simulink library "Renault_Library"  (Simulink 8.2)
settings: Solver=ode3  StartTime=0.0  StopTime=10.0  FixedStep=0.020  RelTol=1e-3
undefined symbols (not defined in the file or in its folder): ABC, AssystemProposal, b, chkval, CoeffA_C, CoeffB_C, CoeffMean_C, ConfTime_C, DiagDelay_C, DividePercent, FailureConfActive_C, FallingEdgeDuration_C, HighLevelThresholdVoltage_C, HighLevelVoltage_C, HighLevelVoltageTunable_C, InitValue_C, InputVoltRangeMax_C, InputVoltRangeMin_C, LowActiveContact_C, LowLevelThresholdVoltage_C, LowLevelVoltage_C, MaxOperatingVoltage_C, MinOperatingVoltage_C, mV2V_conversion, OUT1, OutOfRangeHigh_C, OutOfRangeLow_C, PinSupplySaturationMax, PinSupplySaturationMin, Res1Value_C, Res2Value_C, Res3Value_C, RisingEdgeDuration_C, RSA_CANSignalFailMonitoring, SampleTime_C, service14Table, service19Table, service22Table, service24Table, service28Table, service2ETable, service2FTable, service31Table, service87Table, SizeXStateDID2FTable, SizeXTable14, SizeXTable19, SizeXTable22, SizeXTable24, SizeXTable28, SizeXTable2E, SizeXTable2F, SizeXTable31, SizeXTable87, SizeYStateDID2FTable, SizeYTable14, SizeYTable19, SizeYTable22, SizeYTable24, SizeYTable28 ... (+22)
undefined symbols (not defined in the file): BatteryVoltage_ActivationThreshold_C, FrameStatus_AbsentValue_C, FrameStatus_ConfAbsentValue_C, MemThreshold_C, NAMEOFTHEMODEL_SAMPLE_TIME_CST, NbAbs1_C, NbConfAbs1_C, NbConfPres1_C, NbPres1_C, NOMDUSIGNAL_FaultConfTime_C, NOMDUSIGNAL_FaultRehabTime_C, NOMDUSIGNAL_switchvalue_C, NOMDUSIGNAL_unavailablevalue_C, PeriodFrame1_C, ResetTime_C, WakeUpTime_C
libraries: Renault_Library x112, simulink x75
(part 17 of 32)

== system Renault_Library/Services ECU/Diag/RSA_DiagnosticManagement_v02.00/DIAGNOSTIC_SANCTION_TOP/Service19 ==
  REQ_DATA_NUMBER : Inport
  DIAG_BUFFER_REQUEST : Inport  Port=2
  SESSION_ACTIVE : Inport  Port=3
  Trigger : TriggerPort
  Check_Lenght_Message_Service19 : Subsystem
  Check_Message_Service19 : Stateflow chart  TreatAsAtomicUnit=on
  Parameter_Valid_Service19 : Subsystem
  SubFunction_In_Active_Session_Service19 : Subsystem
  SubFunction_Supported_Service19 : Subsystem
  NEGATIVE_RES_CODE : Outport
  SUPPRESS_POS_RES : Outport  Port=2
  SEND_RES_MESSAGE : Outport  Port=3
  wires:
  REQ_DATA_NUMBER/1 -> Check_Lenght_Message_Service19/1(REQ_DATA_NUMBER)
  Check_Lenght_Message_Service19/1(LENGTH_MESSAGE_CORRECT) -> Check_Message_Service19/1(LENGTH_MESSAGE_CORRECT)
  DIAG_BUFFER_REQUEST/1 -> SubFunction_In_Active_Session_Service19/2(DIAG_BUFFER_REQUEST), Parameter_Valid_Service19/1(DIAG_BUFFER_REQUEST), SubFunction_Supported_Service19/1(DIAG_BUFFER_REQUEST)
  SubFunction_Supported_Service19/1(SUBFUNCTION_SUPPORTED) -> Check_Message_Service19/4(SUBFUNCTION_SUPPORTED)
  SubFunction_Supported_Service19/2(SUPPRESS_POS_RES) -> SUPPRESS_POS_RES/1
  SESSION_ACTIVE/1 -> SubFunction_In_Active_Session_Service19/1(SESSION)
  SubFunction_In_Active_Session_Service19/1(SUBFUNCTION_SUPPORTED_IN_ACTIVE_SESSION) -> Check_Message_Service19/2(SUBFUNCTION_SUPPORTED_IN_ACTIVE_SESSION)
  Parameter_Valid_Service19/1(PARAMETER_VALID) -> Check_Message_Service19/3(PARAMETER_VALID)
  Check_Message_Service19/1(NEGATIVE_RES_CODE) -> NEGATIVE_RES_CODE/1
  Check_Message_Service19/2(SEND_RES_MESSAGE) -> SEND_RES_MESSAGE/1

== system Renault_Library/Services ECU/Diag/RSA_DiagnosticManagement_v02.00/DIAGNOSTIC_SANCTION_TOP/Service22 ==
  REQ_DATA_NUMBER : Inport
  DIAG_BUFFER_REQUEST : Inport  Port=2
  SESSION_ACTIVE : Inport  Port=3
  Trigger : TriggerPort
  Check_Lenght_Message_Response_Service22 : Subsystem
  Check_Lenght_Message_Service22 : Subsystem
  Check_Message_Service22 : Stateflow chart  TreatAsAtomicUnit=on
  Constant : Constant  Value=true  OutDataTypeStr=boolean  SampleTime=-1
  Parameter_Valid_Service22 : Subsystem
  NEGATIVE_RES_CODE : Outport
  LIST_DID_SERVICE_22 : Outport  Port=2  PortDimensions=[2046 3]
  SEND_RES_MESSAGE : Outport  Port=3
  wires:
  Check_Lenght_Message_Service22/1(LENGTH_MESSAGE_CORRECT) -> Check_Message_Service22/1(LENGTH_MESSAGE_CORRECT)
  REQ_DATA_NUMBER/1 -> Check_Lenght_Message_Service22/1(REQ_DATA_NUMBER), Parameter_Valid_Service22/1(REQ_DATA_NUMBER)
  Check_Message_Service22/1(NEGATIVE_RES_CODE) -> NEGATIVE_RES_CODE/1
  DIAG_BUFFER_REQUEST/1 -> Parameter_Valid_Service22/2(DIAG_BUFFER_REQUEST)
  Parameter_Valid_Service22/3(PARAMETER_VALID) -> Check_Message_Service22/5(PARAMETER_VALID)
  Parameter_Valid_Service22/4(LIST_DID_SERVICE_22) -> LIST_DID_SERVICE_22/1
  Constant/1 -> Check_Message_Service22/2(CONDITION_CORRECT)
  Check_Lenght_Message_Response_Service22/1(LENGTH_RESPONSE_CORRECT) -> Check_Message_Service22/3(LENGTH_RESPONSE_CORRECT)
  Parameter_Valid_Service22/1(RESPONSE_SIZE) -> Check_Lenght_Message_Response_Service22/1(RESPONSE_SIZE)
  Parameter_Valid_Service22/2(SECURITY_ACCESS_ACCEPTED) -> Check_Message_Service22/4(SECURITY_ACCESS_ACCEPTED)
  SESSION_ACTIVE/1 -> Parameter_Valid_Service22/3(SESSION_ACTIVE)
  Check_Message_Service22/2(SEND_RES_MESSAGE) -> SEND_RES_MESSAGE/1

== system Renault_Library/Services ECU/Diag/RSA_DiagnosticManagement_v02.00/DIAGNOSTIC_SANCTION_TOP/Service23 ==
  REQ_DATA_NUMBER : Inport
  DIAG_BUFFER_REQUEST : Inport  Port=2
  SESSION_ACTIVE : Inport  Port=3
  Trigger : TriggerPort
  Check_Lenght_Message_Service23 : Subsystem
  Check_Message_Service23 : Stateflow chart  TreatAsAtomicUnit=on
  Constant : Constant  Value=true  OutDataTypeStr=boolean  SampleTime=-1
  Parameter_Valid_Service23 : Subsystem
  NEGATIVE_RES_CODE : Outport
  SEND_RES_MESSAGE : Outport  Port=2
  wires:
  Check_Lenght_Message_Service23/1(LENGTH_MESSAGE_CORRECT) -> Check_Message_Service23/1(LENGTH_MESSAGE_CORRECT)
  REQ_DATA_NUMBER/1 -> Check_Lenght_Message_Service23/1(REQ_DATA_NUMBER), Parameter_Valid_Service23/1(REQ_DATA_NUMBER)
  Check_Message_Service23/1(NEGATIVE_RES_CODE) -> NEGATIVE_RES_CODE/1
  DIAG_BUFFER_REQUEST/1 -> Parameter_Valid_Service23/2(DIAG_BUFFER_REQUEST)
  Constant/1 -> Check_Message_Service23/2(CONDITION_CORRECT)
  SESSION_ACTIVE/1 -> Parameter_Valid_Service23/3(SESSION_ACTIVE)
  Check_Message_Service23/2(SEND_RES_MESSAGE) -> SEND_RES_MESSAGE/1
  Parameter_Valid_Service23/1(SECURITY_ACCESS_ACCEPTED) -> Check_Message_Service23/3(SECURITY_ACCESS_ACCEPTED)
  Parameter_Valid_Service23/2(PARAMETER_VALID) -> Check_Message_Service23/4(PARAMETER_VALID)

== system Renault_Library/Services ECU/Diag/RSA_DiagnosticManagement_v02.00/DIAGNOSTIC_SANCTION_TOP/Service24 ==
  REQ_DATA_NUMBER : Inport
  DIAG_BUFFER_REQUEST : Inport  Port=2
  SESSION_ACTIVE : Inport  Port=3
  Trigger : TriggerPort
  Check_Lenght_Message_Service24 : Subsystem
  Check_Message_Service24 : Stateflow chart  TreatAsAtomicUnit=on
  Constant : Constant  Value=true  OutDataTypeStr=boolean  SampleTime=-1
  Parameter_Valid_Service24 : Subsystem
  NEGATIVE_RES_CODE : Outport
  SEND_RES_MESSAGE : Outport  Port=2
  wires:
  Check_Lenght_Message_Service24/1(LENGTH_MESSAGE_CORRECT) -> Check_Message_Service24/1(LENGTH_MESSAGE_CORRECT)
  REQ_DATA_NUMBER/1 -> Check_Lenght_Message_Service24/1(REQ_DATA_NUMBER)
  Check_Message_Service24/1(NEGATIVE_RES_CODE) -> NEGATIVE_RES_CODE/1
  DIAG_BUFFER_REQUEST/1 -> Parameter_Valid_Service24/1(DIAG_BUFFER_REQUEST)
  Parameter_Valid_Service24/2(PARAMETER_VALID) -> Check_Message_Service24/4(PARAMETER_VALID)
  Constant/1 -> Check_Message_Service24/2(CONDITION_CORRECT)
  Parameter_Valid_Service24/1(SECURITY_ACCESS_ACCEPTED) -> Check_Message_Service24/3(SECURITY_ACCESS_ACCEPTED)
  SESSION_ACTIVE/1 -> Parameter_Valid_Service24/2(SESSION_ACTIVE)
  Check_Message_Service24/2(SEND_RES_MESSAGE) -> SEND_RES_MESSAGE/1

== system Renault_Library/Services ECU/Diag/RSA_DiagnosticManagement_v02.00/DIAGNOSTIC_SANCTION_TOP/Service27 ==
  REQ_DATA_NUMBER : Inport
  DIAG_BUFFER_REQUEST : Inport  Port=2
  SESSION_ACTIVE : Inport  Port=3
  Trigger : TriggerPort
  Check_Lenght_Message_Service27 : Subsystem
  Check_Message_Service27 : Stateflow chart  TreatAsAtomicUnit=on
  Constant{,1..3} (4 alike) : Constant  Value=true  OutDataTypeStr=boolean  SampleTime=-1
  SubFunction_In_Active_Session_Service27 : Subsystem
  SubFunction_Supported_Service27 : Subsystem
  NEGATIVE_RES_CODE : Outport
  SUPPRESS_POS_RES : Outport  Port=2
  SEND_RES_MESSAGE : Outport  Port=3
  wires:
  REQ_DATA_NUMBER/1 -> Check_Lenght_Message_Service27/1(REQ_DATA_NUMBER)
  Check_Lenght_Message_Service27/1(LENGTH_MESSAGE_CORRECT) -> Check_Message_Service27/1(LENGTH_MESSAGE_CORRECT)
  DIAG_BUFFER_REQUEST/1 -> SubFunction_Supported_Service27/1(DIAG_BUFFER_REQUEST), SubFunction_In_Active_Session_Service27/2(DIAG_BUFFER_REQUEST)
  SubFunction_Supported_Service27/1(SUBFUNCTION_SUPPORTED) -> Check_Message_Service27/7(SUBFUNCTION_SUPPORTED)
  SubFunction_Supported_Service27/2(SUPPRESS_POS_RES) -> SUPPRESS_POS_RES/1
  Check_Message_Service27/1(NEGATIVE_RES_CODE) -> NEGATIVE_RES_CODE/1
  SESSION_ACTIVE/1 -> SubFunction_In_Active_Session_Service27/1(SESSION)
  SubFunction_In_Active_Session_Service27/1(SUBFUNCTION_SUPPORTED_IN_ACTIVE_SESSION) -> Check_Message_Service27/6(SUBFUNCTION_SUPPORTED_IN_ACTIVE_SESSION)
  Check_Message_Service27/2(SEND_RES_MESSAGE) -> SEND_RES_MESSAGE/1
  Constant/1 -> Check_Message_Service27/2(CONDITION_CORRECT)
  Constant1/1 -> Check_Message_Service27/3(REQ_SEQUENCE_OK)
  Constant2/1 -> Check_Message_Service27/4(KEY_VALID)
  Constant3/1 -> Check_Message_Service27/5(PARAMETER_VALID)

== system Renault_Library/Services ECU/Diag/RSA_DiagnosticManagement_v02.00/DIAGNOSTIC_SANCTION_TOP/Service28 ==
  REQ_DATA_NUMBER : Inport
  DIAG_BUFFER_REQUEST : Inport  Port=2
  SESSION_ACTIVE : Inport  Port=3
  Trigger : TriggerPort
  Check_Lenght_Message_Service28 : Subsystem
  Check_Message_Service28 : Stateflow chart  TreatAsAtomicUnit=on
  Constant : Constant  Value=true  OutDataTypeStr=boolean  SampleTime=-1
  Parameter_Valid_Service28 : Subsystem
  SubFunction_In_Active_Session_Service28 : Subsystem
  SubFunction_Supported_Service28 : Subsystem
  NEGATIVE_RES_CODE : Outport
  SUPPRESS_POS_RES : Outport  Port=2
  SEND_RES_MESSAGE : Outport  Port=3
  wires:
  REQ_DATA_NUMBER/1 -> Check_Lenght_Message_Service28/1(REQ_DATA_NUMBER)
  DIAG_BUFFER_REQUEST/1 -> SubFunction_Supported_Service28/1(DIAG_BUFFER_OUTPUT), SubFunction_In_Active_Session_Service28/2(DIAG_BUFFER_REQUEST), Parameter_Valid_Service28/1(DIAG_BUFFER_REQUEST)
  SubFunction_Supported_Service28/1(SUBFUNCTION_SUPPORTED) -> Check_Message_Service28/5(SUBFUNCTION_SUPPORTED)
  Check_Message_Service28/1(NEGATIVE_RES_CODE) -> NEGATIVE_RES_CODE/1
  SubFunction_Supported_Service28/2(SUPPRESS_POS_RES) -> SUPPRESS_POS_RES/1
  SubFunction_In_Active_Session_Service28/1(SUBFUNCTION_SUPPORTED_IN_ACTIVE_SESSION) -> Check_Message_Service28/3(SUBFUNCTION_SUPPORTED_IN_ACTIVE_SESSION)
  SESSION_ACTIVE/1 -> SubFunction_In_Active_Session_Service28/1(SESSION)
  Check_Lenght_Message_Service28/1(LENGTH_MESSAGE_CORRECT) -> Check_Message_Service28/1(LENGTH_MESSAGE_CORRECT)
  Check_Message_Service28/2(SEND_RES_MESSAGE) -> SEND_RES_MESSAGE/1
  Parameter_Valid_Service28/1(PARAMETER_VALID) -> Check_Message_Service28/4(PARAMETER_VALID)
  Constant/1 -> Check_Message_Service28/2(CONDITION_CORRECT)

== system Renault_Library/Services ECU/Diag/RSA_DiagnosticManagement_v02.00/DIAGNOSTIC_SANCTION_TOP/Service2E ==
  REQ_DATA_NUMBER : Inport
  DIAG_BUFFER_REQUEST : Inport  Port=2
  SESSION_ACTIVE : Inport  Port=3
  Trigger : TriggerPort
  Check_Lenght_Message_Service2E : Subsystem
  Check_Message_Service2E : Stateflow chart  TreatAsAtomicUnit=on
  Constant : Constant  Value=true  OutDataTypeStr=boolean  SampleTime=-1
  Constant1 : Constant  Value=true  OutDataTypeStr=boolean  SampleTime=-1
  Constant2 : Constant  Value=true  OutDataTypeStr=boolean  SampleTime=-1
  Parameter_Valid_Service2E : Subsystem
  NEGATIVE_RES_CODE : Outport
  SEND_RES_MESSAGE : Outport  Port=2
  wires:
  Constant2/1 -> Check_Message_Service2E/5(PARAMETER_VALID)
  REQ_DATA_NUMBER/1 -> Check_Lenght_Message_Service2E/1(REQ_DATA_NUMBER), Parameter_Valid_Service2E/1(REQ_DATA_NUMBER)
  Check_Lenght_Message_Service2E/1(LENGTH_MESSAGE_CORRECT) -> Check_Message_Service2E/1(LENGTH_MESSAGE_CORRECT)
  Constant/1 -> Check_Message_Service2E/2(CONDITION_CORRECT)
  Constant1/1 -> Check_Message_Service2E/3(CORRECTLY_ALTERED_INTO_MEMORY)
  DIAG_BUFFER_REQUEST/1 -> Parameter_Valid_Service2E/3(DIAG_BUFFER_REQUEST)
  SESSION_ACTIVE/1 -> Parameter_Valid_Service2E/2(SESSION_ACTIVE)
  Parameter_Valid_Service2E/1(SECURITY_ACCESS_ACCEPTED) -> Check_Message_Service2E/4(SECURITY_ACCESS_ACCEPTED)
  Check_Message_Service2E/2(SEND_RES_MESSAGE) -> SEND_RES_MESSAGE/1
  Check_Message_Service2E/1(NEGATIVE_RES_CODE) -> NEGATIVE_RES_CODE/1

== system Renault_Library/Services ECU/Diag/RSA_DiagnosticManagement_v02.00/DIAGNOSTIC_SANCTION_TOP/Service2F ==
  REQ_DATA_NUMBER : Inport
  SESSION_ACTIVE : Inport  Port=2
  DIAG_BUFFER_REQUEST : Inport  Port=3
  Trigger : TriggerPort
  Check_Lenght_Message_Service2F : Subsystem
  Check_Message_Service2F : Stateflow chart  TreatAsAtomicUnit=on
  Constant : Constant  Value=true  OutDataTypeStr=boolean  SampleTime=-1
  Parameter_Valid_Service2F : Subsystem
  NEGATIVE_RES_CODE : Outport
  SEND_RES_MESSAGE : Outport  Port=2
  wires:
  REQ_DATA_NUMBER/1 -> Check_Lenght_Message_Service2F/1(REQ_DATA_NUMBER)
  Check_Lenght_Message_Service2F/1(LENGTH_MESSAGE_CORRECT) -> Check_Message_Service2F/1(LENGTH_MESSAGE_CORRECT)
  Check_Message_Service2F/1(NEGATIVE_RES_CODE) -> NEGATIVE_RES_CODE/1
  Check_Message_Service2F/2(SEND_RES_MESSAGE) -> SEND_RES_MESSAGE/1
  Parameter_Valid_Service2F/2(PARAMETER_VALID) -> Check_Message_Service2F/4(PARAMETER_VALID)
  SESSION_ACTIVE/1 -> Parameter_Valid_Service2F/1(SESSION_ACTIVE)
  DIAG_BUFFER_REQUEST/1 -> Parameter_Valid_Service2F/2(DIAG_BUFFER_REQUEST)
  Parameter_Valid_Service2F/1(SECURITY_ACCESS_ACCEPTED) -> Check_Message_Service2F/3(SECURITY_ACCESS_ACCEPTED)
  Constant/1 -> Check_Message_Service2F/2(CONDITION_CORRECT)

== system Renault_Library/Services ECU/Diag/RSA_DiagnosticManagement_v02.00/DIAGNOSTIC_SANCTION_TOP/Service31 ==
  REQ_DATA_NUMBER : Inport
  DIAG_BUFFER_REQUEST : Inport  Port=2
  SESSION_ACTIVE : Inport  Port=3
  Trigger : TriggerPort
  Check_Lenght_Message_Service31 : Subsystem
  Check_Message_Service31 : Stateflow chart  TreatAsAtomicUnit=on
  Constant : Constant  Value=true  OutDataTypeStr=boolean  SampleTime=-1
  Constant1 : Constant  Value=true  OutDataTypeStr=boolean  SampleTime=-1
  Parameter_Valid_Service31 : Subsystem
  SubFunction_In_Active_Session_Service31 : Subsystem
  SubFunction_Supported_Service31 : Subsystem
  NEGATIVE_RES_CODE : Outport
  SUPPRESS_POS_RES : Outport  Port=2
  SEND_RES_MESSAGE : Outport  Port=3
  wires:
  REQ_DATA_NUMBER/1 -> Check_Lenght_Message_Service31/1(REQ_DATA_NUMBER)
  Check_Lenght_Message_Service31/1(LENGTH_MESSAGE_CORRECT) -> Check_Message_Service31/1(LENGTH_MESSAGE_CORRECT)
  Check_Message_Service31/1(NEGATIVE_RES_CODE) -> NEGATIVE_RES_CODE/1
  SubFunction_Supported_Service31/1(SUBFUNCTION_SUPPORTED) -> Check_Message_Service31/7(SUBFUNCTION_SUPPORTED)
  DIAG_BUFFER_REQUEST/1 -> SubFunction_In_Active_Session_Service31/2(DIAG_BUFFER_REQUEST), SubFunction_Supported_Service31/1(DIAG_BUFFER_REQUEST), [DIAG_BUFFER_REQUEST]
  SubFunction_Supported_Service31/2(SUPPRESS_POS_RES) -> SUPPRESS_POS_RES/1
  SubFunction_In_Active_Session_Service31/1(SUBFUNCTION_SUPPORTED_IN_ACTIVE_SESSION) -> Check_Message_Service31/6(SUBFUNCTION_SUPPORTED_IN_ACTIVE_SESSION)
  SESSION_ACTIVE/1 -> SubFunction_In_Active_Session_Service31/1(SESSION), Parameter_Valid_Service31/2(SESSION_ACTIVE)
  Check_Message_Service31/2(SEND_RES_MESSAGE) -> SEND_RES_MESSAGE/1
  [DIAG_BUFFER_REQUEST] -> Parameter_Valid_Service31/1(DIAG_BUFFER_REQUEST)
  Parameter_Valid_Service31/1(SECURITY_ACCESS_ACCEPTED) -> Check_Message_Service31/4(SECURITY_ACCESS_ACCEPTED)
  Parameter_Valid_Service31/2(PARAMETER_VALID) -> Check_Message_Service31/5(PARAMETER_VALID)
  Constant/1 -> Check_Message_Service31/2(REQ_SEQUENCE_OK)
  Constant1/1 -> Check_Message_Service31/3(CONDITION_CORRECT)

== system Renault_Library/Services ECU/Diag/RSA_DiagnosticManagement_v02.00/DIAGNOSTIC_SANCTION_TOP/Service3D ==
  REQ_DATA_NUMBER : Inport
  DIAG_BUFFER_REQUEST : Inport  Port=2
  SESSION_ACTIVE : Inport  Port=3
  Trigger : TriggerPort
  Check_Lenght_Message_Service3D : Subsystem
  Check_Message_Service3D : Stateflow chart  TreatAsAtomicUnit=on
  Constant : Constant  Value=true  OutDataTypeStr=boolean  SampleTime=-1
  Constant1 : Constant  Value=true  OutDataTypeStr=boolean  SampleTime=-1
  Parameter_Valid_Service3D : Subsystem
  NEGATIVE_RES_CODE : Outport
  SEND_RES_MESSAGE : Outport  Port=2
  wires:
  Check_Message_Service3D/1(NEGATIVE_RES_CODE) -> NEGATIVE_RES_CODE/1
  Check_Message_Service3D/2(SEND_RES_MESSAGE) -> SEND_RES_MESSAGE/1
  SESSION_ACTIVE/1 -> Parameter_Valid_Service3D/2(SESSION_ACTIVE)
  DIAG_BUFFER_REQUEST/1 -> Parameter_Valid_Service3D/3(DIAG_BUFFER_REQUEST)
  Constant1/1 -> Check_Message_Service3D/3(CORRECTLY_ALTERED_INTO_MEMORY)
  Constant/1 -> Check_Message_Service3D/2(CONDITION_CORRECT)
  Check_Lenght_Message_Service3D/1(LENGTH_MESSAGE_CORRECT) -> Check_Message_Service3D/1(LENGTH_MESSAGE_CORRECT)
  REQ_DATA_NUMBER/1 -> Parameter_Valid_Service3D/1(REQ_DATA_NUMBER), Check_Lenght_Message_Service3D/1(REQ_DATA_NUMBER)
  Parameter_Valid_Service3D/1(SECURITY_ACCESS_ACCEPTED) -> Check_Message_Service3D/4(SECURITY_ACCESS_ACCEPTED)
  Parameter_Valid_Service3D/2(PARAMETER_VALID) -> Check_Message_Service3D/5(PARAMETER_VALID)

== system Renault_Library/Services ECU/Diag/RSA_DiagnosticManagement_v02.00/DIAGNOSTIC_SANCTION_TOP/Service3E ==
  REQ_DATA_NUMBER : Inport
  DIAG_BUFFER_REQUEST : Inport  Port=2
  SESSION_ACTIVE : Inport  Port=3
  Trigger : TriggerPort
  Check_Lenght_Message_Service3E : Subsystem
  Check_Message_Service3E : Stateflow chart  TreatAsAtomicUnit=on
  SubFunction_In_Active_Session_Service3E : Subsystem
  SubFunction_Supported_Service3E : Subsystem
  SUPPRESS_POS_RES : Outport
  NEGATIVE_RES_CODE : Outport  Port=2
  SEND_RES_MESSAGE : Outport  Port=3
  wires:
  SubFunction_Supported_Service3E/1(SUBFUNCTION_SUPPORTED) -> Check_Message_Service3E/3(SUBFUNCTION_SUPPORTED)
  REQ_DATA_NUMBER/1 -> Check_Lenght_Message_Service3E/1(REQ_DATA_NUMBER)
  Check_Lenght_Message_Service3E/1(LENGTH_MESSAGE_CORRECT) -> Check_Message_Service3E/1(LENGTH_MESSAGE_CORRECT)
  SubFunction_Supported_Service3E/2(SUPPRESS_POS_RES) -> SUPPRESS_POS_RES/1
  SESSION_ACTIVE/1 -> SubFunction_In_Active_Session_Service3E/1(SESSION)
  SubFunction_In_Active_Session_Service3E/1(SUBFUNCTION_SUPPORTED_IN_ACTIVE_SESSION) -> Check_Message_Service3E/2(SUBFUNCTION_SUPPORTED_IN_ACTIVE_SESSION)
  DIAG_BUFFER_REQUEST/1 -> SubFunction_In_Active_Session_Service3E/2(DIAG_BUFFER_REQUEST), SubFunction_Supported_Service3E/1(DIAG_BUFFER_REQUEST)
  Check_Message_Service3E/2(SEND_RES_MESSAGE) -> SEND_RES_MESSAGE/1
  Check_Message_Service3E/1(NEGATIVE_RES_CODE) -> NEGATIVE_RES_CODE/1

== system Renault_Library/Services ECU/Diag/RSA_DiagnosticManagement_v02.00/DIAGNOSTIC_SANCTION_TOP/Service87 ==
  REQ_DATA_NUMBER : Inport
  DIAG_BUFFER_REQUEST : Inport  Port=2
  SESSION_ACTIVE : Inport  Port=3
  Trigger : TriggerPort
  Check_Lenght_Message_Service87 : Subsystem
  Check_Message_Service87 : Stateflow chart  TreatAsAtomicUnit=on
  Constant : Constant  Value=true  OutDataTypeStr=boolean  SampleTime=-1
  Constant1 : Constant  Value=true  OutDataTypeStr=boolean  SampleTime=-1
  Parameter_Valid_Service87 : Subsystem
  SubFunction_In_Active_Session_Service87 : Subsystem
  SubFunction_Supported_Service87 : Subsystem
  NEGATIVE_RES_CODE : Outport
  SUPPRESS_POS_RES : Outport  Port=2
  SEND_RES_MESSAGE : Outport  Port=3
  wires:
  Parameter_Valid_Service87/1(PARAMETER_VALID) -> Check_Message_Service87/5(PARAMETER_VALID)
  Constant1/1 -> Check_Message_Service87/3(REQ_SEQUENCE_OK)
  Constant/1 -> Check_Message_Service87/2(CONDITION_CORRECT)
  Check_Message_Service87/2(SEND_RES_MESSAGE) -> SEND_RES_MESSAGE/1
  SubFunction_In_Active_Session_Service87/1(SUBFUNCTION_SUPPORTED_IN_ACTIVE_SESSION) -> Check_Message_Service87/4(SUBFUNCTION_SUPPORTED_IN_ACTIVE_SESSION)
  SubFunction_Supported_Service87/2(SUPPRESS_POS_RES) -> SUPPRESS_POS_RES/1
  DIAG_BUFFER_REQUEST/1 -> SubFunction_Supported_Service87/1(DIAG_BUFFER_REQUEST), SubFunction_In_Active_Session_Service87/2(DIAG_BUFFER_REQUEST), Parameter_Valid_Service87/1(DIAG_BUFFER_REQUEST)
  SubFunction_Supported_Service87/1(SUBFUNCTION_SUPPORTED) -> Check_Message_Service87/6(SUBFUNCTION_SUPPORTED)
  Check_Message_Service87/1(NEGATIVE_RES_CODE) -> NEGATIVE_RES_CODE/1
  Check_Lenght_Message_Service87/1(LENGTH_MESSAGE_CORRECT) -> Check_Message_Service87/1(LENGTH_MESSAGE_CORRECT)
  REQ_DATA_NUMBER/1 -> Check_Lenght_Message_Service87/1(REQ_DATA_NUMBER)
  SESSION_ACTIVE/1 -> SubFunction_In_Active_Session_Service87/1(SESSION)

== system Renault_Library/Services ECU/Diag/RSA_DiagnosticManagement_v02.00/DIAGNOSTIC_SANCTION_TOP/SessionDiagnostic ==
  DIAG_BUFFER_REQUEST : Inport
  Diagnostic_Session_Control : Stateflow chart  TreatAsAtomicUnit=on
  Request_Of_Session : Stateflow chart  TreatAsAtomicUnit=on
  Tempo_Tester_Present : Stateflow chart  TreatAsAtomicUnit=on
  SESSION_ACTIVE : Outport  OutDataTypeStr=Enum: TYP_SESSION_ACTIVE
  tag scope: EV_TESTER_PRESENT=global
  wires:
  Request_Of_Session/1(Session) -> Diagnostic_Session_Control/2(Session)
  Diagnostic_Session_Control/1(SESSION_ACTIVE) -> SESSION_ACTIVE/1
  Tempo_Tester_Present/1(EV_TESTER_PRESENT) -> [EV_TESTER_PRESENT]
  [EV_TESTER_PRESENT] -> Diagnostic_Session_Control/1(EV_TESTER_PRESENT)
  DIAG_BUFFER_REQUEST/1 -> [DIAG_BUFFER_REQUEST]
  [DIAG_BUFFER_REQUEST] -> Request_Of_Session/1(DIAG_BUFFER_REQUEST)
  [DIAG_BUFFER_REQUEST] -> Tempo_Tester_Present/1(DIAG_BUFFER_REQUEST)

== system Renault_Library/Services ECU/Diag/RSA_DiagnosticManagement_v02.00/DIAGNOSTIC_SEGMENTATION_TOP/Frame_Type ==
  DTOOL_TO_ECU : Inport
  BusSelector3 : BusSelector  OutputSignals=DTOOL_TO_ECU_PART1.DTOOL_TO_ECU_BYTE_0
  ShiftArithmetic : ArithShift  BitShiftNumber=4
  REQ_FRAME_TYPE : Outport  OutDataTypeStr=uint8
  wires:
  BusSelector3/1 -> ShiftArithmetic/1  "<DTOOL_TO_ECU_BYTE_0>"
  DTOOL_TO_ECU/1 -> BusSelector3/1  "DTOOL_TO_ECU"
  ShiftArithmetic/1 -> REQ_FRAME_TYPE/1

== system Renault_Library/Services ECU/Diag/RSA_DiagnosticManagement_v02.00/DIAGNOSTIC_SEGMENTATION_TOP/Request_Frame ==
  PART1_DTOOL_TO_ECU : Inport
  PART2_DTOOL_TO_ECU : Inport  Port=2
  BitwiseOperator{,1..7} (8 alike) : simulink/Logic and Bit Operations/Bitwise Operator  logicop=AND  UseBitMask=on  BitMask=bin2dec('11111111')  BitMaskRealWorld=Stored Integer
  BusCreator : BusCreator
  BusCreator1 : BusCreator
  BusCreator2 : BusCreator  Inputs=2
  DataTypeConversion{,1..7} (8 alike) : DataTypeConversion  OutDataTypeStr=uint8  ConvertRealWorld=Stored Integer (SI)  RndMeth=Zero
  ShiftArithmetic{,1..5} (6 alike) : ArithShift  BitShiftNumber=8
  DTOOL_TO_ECU : Outport  OutDataTypeStr=uint8  PortDimensions=8
  wires:
  DataTypeConversion1/1 -> BusCreator1/2  "DTOOL_TO_ECU_BYTE_1"
  DataTypeConversion2/1 -> BusCreator1/3  "DTOOL_TO_ECU_BYTE_2"
  DataTypeConversion3/1 -> BusCreator1/4  "DTOOL_TO_ECU_BYTE_3"
  DataTypeConversion4/1 -> BusCreator/1  "DTOOL_TO_ECU_BYTE_4"
  DataTypeConversion5/1 -> BusCreator/2  "DTOOL_TO_ECU_BYTE_5"
  DataTypeConversion6/1 -> BusCreator/3  "DTOOL_TO_ECU_BYTE_6"
  BusCreator2/1 -> DTOOL_TO_ECU/1  "DTOOL_TO_ECU"
  DataTypeConversion/1 -> BusCreator1/1  "DTOOL_TO_ECU_BYTE_0"
  PART1_DTOOL_TO_ECU/1 -> ShiftArithmetic/1, BitwiseOperator3/1
  ShiftArithmetic/1 -> ShiftArithmetic1/1, BitwiseOperator2/1
  ShiftArithmetic1/1 -> ShiftArithmetic2/1, BitwiseOperator1/1
  ShiftArithmetic2/1 -> BitwiseOperator/1
  BitwiseOperator/1 -> DataTypeConversion/1
  BitwiseOperator1/1 -> DataTypeConversion1/1
  BitwiseOperator2/1 -> DataTypeConversion2/1
  BitwiseOperator3/1 -> DataTypeConversion3/1
  PART2_DTOOL_TO_ECU/1 -> ShiftArithmetic3/1, BitwiseOperator7/1
  ShiftArithmetic3/1 -> ShiftArithmetic4/1, BitwiseOperator6/1
  ShiftArithmetic4/1 -> ShiftArithmetic5/1, BitwiseOperator5/1
  ShiftArithmetic5/1 -> BitwiseOperator4/1
  BusCreator/1 -> BusCreator2/2  "DTOOL_TO_ECU_PART2"
  BusCreator1/1 -> BusCreator2/1  "DTOOL_TO_ECU_PART1"
  BitwiseOperator4/1 -> DataTypeConversion4/1
  BitwiseOperator5/1 -> DataTypeConversion5/1
  BitwiseOperator6/1 -> DataTypeConversion6/1
  BitwiseOperator7/1 -> DataTypeConversion7/1
  DataTypeConversion7/1 -> BusCreator/4  "DTOOL_TO_ECU_BYTE_7"

== system Renault_Library/Services ECU/Diag/RSA_DiagnosticManagement_v02.00/DIAGNOSTIC_SEGMENTATION_TOP/Response_Frame ==
  ECU_TO_DTOOL : Inport  OutDataTypeStr=uint8  PortDimensions=8
  BusSelector1 : BusSelector  OutputSignals=ECU_TO_DTOOL_BYTE_0,ECU_TO_DTOOL_BYTE_1,ECU_TO_DTOOL_BYTE_2,ECU_TO_DTOOL_BYTE_3,ECU_TO_DTOOL_BYTE_4,ECU_TO_DTOOL_BYTE_5,ECU_TO_DTOOL_BYTE_6,ECU_TO_DTOOL_BYTE_7
  DataTypeConversion{1..8} (8 alike) : DataTypeConversion  OutDataTypeStr=uint32  ConvertRealWorld=Stored Integer (SI)  RndMeth=Zero
  ShiftArithmetic{,1..5} (6 alike) : ArithShift  BitShiftNumber=-8
  Sum{1..5,8} (6 alike) : Sum  OutDataTypeStr=Inherit: Inherit via back propagation  RndMeth=Zero  InputSameDT=on
  PART1_ECU_TO_DTOOL : Outport  OutDataTypeStr=uint32
  PART2_ECU_TO_DTOOL : Outport  Port=2  OutDataTypeStr=uint32
  wires:
  BusSelector1/1 -> DataTypeConversion7/1  "<ECU_TO_DTOOL_BYTE_0>"
  ShiftArithmetic/1 -> Sum8/1
  BusSelector1/2 -> DataTypeConversion1/1  "<ECU_TO_DTOOL_BYTE_1>"
  ShiftArithmetic1/1 -> Sum1/1
  BusSelector1/3 -> DataTypeConversion3/1  "<ECU_TO_DTOOL_BYTE_2>"
  Sum1/1 -> ShiftArithmetic2/1
  BusSelector1/4 -> DataTypeConversion2/1  "<ECU_TO_DTOOL_BYTE_3>"
  Sum2/1 -> PART1_ECU_TO_DTOOL/1
  BusSelector1/5 -> DataTypeConversion8/1  "<ECU_TO_DTOOL_BYTE_4>"
  ShiftArithmetic3/1 -> Sum3/1
  BusSelector1/6 -> DataTypeConversion4/1  "<ECU_TO_DTOOL_BYTE_5>"
  Sum3/1 -> ShiftArithmetic4/1
  ShiftArithmetic4/1 -> Sum4/1
  BusSelector1/7 -> DataTypeConversion6/1  "<ECU_TO_DTOOL_BYTE_6>"
  Sum4/1 -> ShiftArithmetic5/1
  BusSelector1/8 -> DataTypeConversion5/1  "<ECU_TO_DTOOL_BYTE_7>"
  ShiftArithmetic5/1 -> Sum5/1
  Sum5/1 -> PART2_ECU_TO_DTOOL/1
  DataTypeConversion8/1 -> ShiftArithmetic3/1
  DataTypeConversion4/1 -> Sum3/2
  DataTypeConversion6/1 -> Sum4/2
  DataTypeConversion2/1 -> Sum2/2
  DataTypeConversion3/1 -> Sum1/2
  DataTypeConversion1/1 -> Sum8/2
  DataTypeConversion7/1 -> ShiftArithmetic/1
  ECU_TO_DTOOL/1 -> BusSelector1/1  "ECU_TO_DTOOL"
  Sum8/1 -> ShiftArithmetic1/1
  ShiftArithmetic2/1 -> Sum2/1
  DataTypeConversion5/1 -> Sum5/2

== system Renault_Library/Services ECU/Diag/RSA_DiagnosticManagement/DIAGNOSTIC_SANCTION_TOP/GeneralService/Server_State ==
  SEG_STATE : Inport
  Constant : Constant  Value=TYP_SEG_STATE.TRANSMIT_IN_PROGRESS  OutDataTypeStr=Enum: TYP_SEG_STATE  SampleTime=-1
  RelationalOperator : RelationalOperator  Operator=~=  OutDataTypeStr=boolean  InputSameDT=on
  ECU_AVAILABLE : Outport
  wires:
  Constant/1 -> RelationalOperator/2
  SEG_STATE/1 -> RelationalOperator/1
  RelationalOperator/1 -> ECU_AVAILABLE/1

== system Renault_Library/Services ECU/Diag/RSA_DiagnosticManagement/DIAGNOSTIC_SANCTION_TOP/GeneralService/Service_Supported ==
  DIAG_BUFFER_REQUEST : Inport
  Constant : Constant  Value=Service10  OutDataTypeStr=Inherit: Inherit via back propagation  SampleTime=-1
  Constant{1..2,4..5,7,9,11..13,15..16,18..19,21,23..25,27..29,31..32,34..36} (25 alike) : Constant  Value=true  OutDataTypeStr=boolean  SampleTime=-1
  Constant10 : Constant  Value=CommunicationControl  OutDataTypeStr=Inherit: Inherit via back propagation  SampleTime=-1
  Constant14 : Constant  Value=ControlDTCSetting  OutDataTypeStr=Inherit: Inherit via back propagation  SampleTime=-1
  Constant17 : Constant  Value=TesterPresent  OutDataTypeStr=Inherit: Inherit via back propagation  SampleTime=-1
  Constant20 : Constant  Value=AccessTimingParameter  OutDataTypeStr=Inherit: Inherit via back propagation  SampleTime=-1
  Constant22 : Constant  Value=SecuredDataTransmission  OutDataTypeStr=Inherit: Inherit via back propagation  SampleTime=-1
  Constant26 : Constant  Value=ReadMemoryByAddress  OutDataTypeStr=Inherit: Inherit via back propagation  SampleTime=-1
  Constant3 : Constant  Value=DiagnosticSessionControl  OutDataTypeStr=Inherit: Inherit via back propagation  SampleTime=-1
  Constant30 : Constant  Value=WriteDataByIdentifier  OutDataTypeStr=Inherit: Inherit via back propagation  SampleTime=-1
  Constant33 : Constant  Value=ReadScalingDataByIdentifier  OutDataTypeStr=Inherit: Inherit via back propagation  SampleTime=-1
  Constant37 : Constant  Value=ReadDataByPeriodicIdentifier  OutDataTypeStr=Inherit: Inherit via back propagation  SampleTime=-1
  Constant39 : Constant  Value=DynamicallyDefineDataIdentifier  OutDataTypeStr=Inherit: Inherit via back propagation  SampleTime=-1
  Constant41 : Constant  Value=ResponseOnEvent  OutDataTypeStr=Inherit: Inherit via back propagation  SampleTime=-1
  Constant44 : Constant  Value=LinControl  OutDataTypeStr=Inherit: Inherit via back propagation  SampleTime=-1
  Constant46 : Constant  Value=ReadDataByIdentifier  OutDataTypeStr=Inherit: Inherit via back propagation  SampleTime=-1
  Constant50 : Constant  Value=InputOutputConrolByIdentifier  OutDataTypeStr=Inherit: Inherit via back propagation  SampleTime=-1
  Constant54 : Constant  Value=TransfertData  OutDataTypeStr=Inherit: Inherit via back propagation  SampleTime=-1
  Constant57 : Constant  Value=RoutineControl  OutDataTypeStr=Inherit: Inherit via back propagation  SampleTime=-1
  Constant6 : Constant  Value=EcuReset  OutDataTypeStr=Inherit: Inherit via back propagation  SampleTime=-1
  Constant61 : Constant  Value=RequestDownload  OutDataTypeStr=Inherit: Inherit via back propagation  SampleTime=-1
  Constant63 : Constant  Value=RequestUpload  OutDataTypeStr=Inherit: Inherit via back propagation  SampleTime=-1
  Constant66 : Constant  Value=Service11  OutDataTypeStr=Inherit: Inherit via back propagation  SampleTime=-1
  Constant67 : Constant  Value=Service27  OutDataTypeStr=Inherit: Inherit via back propagation  SampleTime=-1
  Constant68 : Constant  Value=Service28  OutDataTypeStr=Inherit: Inherit via back propagation  SampleTime=-1
  Constant69 : Constant  Value=Service3E  OutDataTypeStr=Inherit: Inherit via back propagation  SampleTime=-1
  Constant70 : Constant  Value=Service83  OutDataTypeStr=Inherit: Inherit via back propagation  SampleTime=-1
  Constant71 : Constant  Value=WriteDataByAddress  OutDataTypeStr=Inherit: Inherit via back propagation  SampleTime=-1
  Constant72 : Constant  Value=Service84  OutDataTypeStr=Inherit: Inherit via back propagation  SampleTime=-1
  Constant73 : Constant  Value=Service85  OutDataTypeStr=Inherit: Inherit via back propagation  SampleTime=-1
  Constant74 : Constant  Value=Service86  OutDataTypeStr=Inherit: Inherit via back propagation  SampleTime=-1
  Constant75 : Constant  Value=Service87  OutDataTypeStr=Inherit: Inherit via back propagation  SampleTime=-1
  Constant76 : Constant  Value=Service22  OutDataTypeStr=Inherit: Inherit via back propagation  SampleTime=-1
  Constant77 : Constant  Value=Service23  OutDataTypeStr=Inherit: Inherit via back propagation  SampleTime=-1
  Constant78 : Constant  Value=Service24  OutDataTypeStr=Inherit: Inherit via back propagation  SampleTime=-1
  Constant79 : Constant  Value=Service2A  OutDataTypeStr=Inherit: Inherit via back propagation  SampleTime=-1
  Constant8 : Constant  Value=SecurityAccess  OutDataTypeStr=Inherit: Inherit via back propagation  SampleTime=-1
  Constant80 : Constant  Value=Service2C  OutDataTypeStr=Inherit: Inherit via back propagation  SampleTime=-1
  Constant81 : Constant  Value=Service2E  OutDataTypeStr=Inherit: Inherit via back propagation  SampleTime=-1
  Constant83 : Constant  Value=Service3D  OutDataTypeStr=Inherit: Inherit via back propagation  SampleTime=-1
  Constant84 : Constant  Value=RequestTransfertExit  OutDataTypeStr=Inherit: Inherit via back propagation  SampleTime=-1
  Constant85 : Constant  Value=Service14  OutDataTypeStr=Inherit: Inherit via back propagation  SampleTime=-1
  Constant87 : Constant  Value=Service19  OutDataTypeStr=Inherit: Inherit via back propagation  SampleTime=-1
  Constant88 : Constant  Value=Service2F  OutDataTypeStr=Inherit: Inherit via back propagation  SampleTime=-1
  Constant89 : Constant  Value=Service31  OutDataTypeStr=Inherit: Inherit via back propagation  SampleTime=-1
  Constant90 : Constant  Value=Service34  OutDataTypeStr=Inherit: Inherit via back propagation  SampleTime=-1
  Constant92 : Constant  Value=ClearDiagnosticInformation  OutDataTypeStr=Inherit: Inherit via back propagation  SampleTime=-1
  Constant94 : Constant  Value=ReadDTCInformation  OutDataTypeStr=Inherit: Inherit via back propagation  SampleTime=-1
  Constant96 : Constant  Value=Service35  OutDataTypeStr=Inherit: Inherit via back propagation  SampleTime=-1
  Constant97 : Constant  Value=Service36  OutDataTypeStr=Inherit: Inherit via back propagation  SampleTime=-1
  Constant98 : Constant  Value=Service37  OutDataTypeStr=Inherit: Inherit via back propagation  SampleTime=-1
  LogicalOperator{,1..3,5..18,26..32} (25 alike) : Logic  OutDataTypeStr=boolean
  LogicalOperator4 : Logic  Operator=OR  Inputs=25  OutDataTypeStr=boolean
  RelationalOperator{,1..40,44,51,55,58..63} (50 alike) : RelationalOperator  Operator===  OutDataTypeStr=boolean  InputSameDT=on
  Selector : Selector  InputPortWidth=4095  IndexOptions=Index vector (dialog)  Indices=[1]  OutputSizes=1
  SERVICE_SUPPORTED : Outport
  wires:
  DIAG_BUFFER_REQUEST/1 -> Selector/1
  Constant/1 -> RelationalOperator/2
  Constant66/1 -> RelationalOperator1/2
  Constant67/1 -> RelationalOperator2/2
  Constant68/1 -> RelationalOperator3/2
  Constant3/1 -> RelationalOperator4/1
  Constant1/1 -> RelationalOperator4/2
  RelationalOperator/1 -> LogicalOperator/2
  RelationalOperator4/1 -> LogicalOperator/1
  Constant2/1 -> RelationalOperator5/2
  Constant6/1 -> RelationalOperator5/1
  RelationalOperator1/1 -> LogicalOperator1/2
  RelationalOperator5/1 -> LogicalOperator1/1
  Constant4/1 -> RelationalOperator6/2
  Constant8/1 -> RelationalOperator6/1
  RelationalOperator2/1 -> LogicalOperator2/2
  RelationalOperator6/1 -> LogicalOperator2/1
  Constant10/1 -> RelationalOperator7/1
  Constant5/1 -> RelationalOperator7/2
  RelationalOperator7/1 -> LogicalOperator3/1
  RelationalOperator3/1 -> LogicalOperator3/2
  Constant69/1 -> RelationalOperator8/2
  Constant70/1 -> RelationalOperator9/2
  Constant72/1 -> RelationalOperator10/2
  Constant73/1 -> RelationalOperator11/2
  Constant17/1 -> RelationalOperator12/1
  Constant7/1 -> RelationalOperator12/2
  RelationalOperator8/1 -> LogicalOperator5/2
  RelationalOperator12/1 -> LogicalOperator5/1
  Constant9/1 -> RelationalOperator13/2
  Constant20/1 -> RelationalOperator13/1
  RelationalOperator9/1 -> LogicalOperator6/2
  RelationalOperator13/1 -> LogicalOperator6/1
  Constant11/1 -> RelationalOperator14/2
  Constant22/1 -> RelationalOperator14/1
  RelationalOperator10/1 -> LogicalOperator7/2
  RelationalOperator14/1 -> LogicalOperator7/1
  Constant14/1 -> RelationalOperator15/1
  Constant12/1 -> RelationalOperator15/2
  RelationalOperator15/1 -> LogicalOperator8/1
  RelationalOperator11/1 -> LogicalOperator8/2
  Constant74/1 -> RelationalOperator16/2
  Constant75/1 -> RelationalOperator17/2
  Constant76/1 -> RelationalOperator24/2
  Constant77/1 -> RelationalOperator25/2
  Constant41/1 -> RelationalOperator26/1
  Constant13/1 -> RelationalOperator26/2
  RelationalOperator16/1 -> LogicalOperator9/2
  RelationalOperator26/1 -> LogicalOperator9/1
  Constant15/1 -> RelationalOperator27/2
  Constant44/1 -> RelationalOperator27/1
  RelationalOperator17/1 -> LogicalOperator10/2
  RelationalOperator27/1 -> LogicalOperator10/1
  Constant16/1 -> RelationalOperator28/2
  Constant46/1 -> RelationalOperator28/1
  RelationalOperator24/1 -> LogicalOperator11/2
  RelationalOperator28/1 -> LogicalOperator11/1
  Constant26/1 -> RelationalOperator29/1
  Constant18/1 -> RelationalOperator29/2
  RelationalOperator29/1 -> LogicalOperator12/1
  RelationalOperator25/1 -> LogicalOperator12/2
  Constant78/1 -> RelationalOperator30/2
  Constant79/1 -> RelationalOperator31/2
  Constant80/1 -> RelationalOperator18/2
  Constant81/1 -> RelationalOperator19/2
  Constant33/1 -> RelationalOperator20/1
  Constant19/1 -> RelationalOperator20/2
  RelationalOperator30/1 -> LogicalOperator13/2
  RelationalOperator20/1 -> LogicalOperator13/1
  Constant21/1 -> RelationalOperator21/2
  Constant37/1 -> RelationalOperator21/1
  RelationalOperator31/1 -> LogicalOperator14/2
  RelationalOperator21/1 -> LogicalOperator14/1
  Constant23/1 -> RelationalOperator22/2
  Constant39/1 -> RelationalOperator22/1
  RelationalOperator18/1 -> LogicalOperator15/2
  RelationalOperator22/1 -> LogicalOperator15/1
  Constant30/1 -> RelationalOperator23/1
  Constant24/1 -> RelationalOperator23/2
  RelationalOperator23/1 -> LogicalOperator16/1
  RelationalOperator19/1 -> LogicalOperator16/2
  Constant83/1 -> RelationalOperator32/2
  Constant85/1 -> RelationalOperator33/2
  Constant87/1 -> RelationalOperator44/2
  Constant88/1 -> RelationalOperator55/2
  Constant71/1 -> RelationalOperator58/1
  Constant25/1 -> RelationalOperator58/2
  RelationalOperator32/1 -> LogicalOperator17/2
  RelationalOperator58/1 -> LogicalOperator17/1
  Constant27/1 -> RelationalOperator59/2
  Constant92/1 -> RelationalOperator59/1
  RelationalOperator33/1 -> LogicalOperator18/2
  RelationalOperator59/1 -> LogicalOperator18/1
  Constant28/1 -> RelationalOperator60/2
  Constant94/1 -> RelationalOperator60/1
  RelationalOperator44/1 -> LogicalOperator26/2
  RelationalOperator60/1 -> LogicalOperator26/1
  Constant50/1 -> RelationalOperator61/1
  Constant29/1 -> RelationalOperator61/2
  RelationalOperator61/1 -> LogicalOperator27/1
  RelationalOperator55/1 -> LogicalOperator27/2
  Constant89/1 -> RelationalOperator62/2
  Constant90/1 -> RelationalOperator63/2
  Constant96/1 -> RelationalOperator34/2
  Constant97/1 -> RelationalOperator35/2
  Constant57/1 -> RelationalOperator36/1
  Constant31/1 -> RelationalOperator36/2
  RelationalOperator62/1 -> LogicalOperator28/2
  RelationalOperator36/1 -> LogicalOperator28/1
  Constant32/1 -> RelationalOperator37/2
  Constant61/1 -> RelationalOperator37/1
  RelationalOperator63/1 -> LogicalOperator29/2
  RelationalOperator37/1 -> LogicalOperator29/1
  Constant34/1 -> RelationalOperator38/2
  Constant63/1 -> RelationalOperator38/1
  RelationalOperator34/1 -> LogicalOperator30/2
  RelationalOperator38/1 -> LogicalOperator30/1
  Constant54/1 -> RelationalOperator39/1
  Constant35/1 -> RelationalOperator39/2
  RelationalOperator39/1 -> LogicalOperator31/1
  RelationalOperator35/1 -> LogicalOperator31/2
  Constant98/1 -> RelationalOperator40/2
  Constant84/1 -> RelationalOperator51/1
  Constant36/1 -> RelationalOperator51/2
  RelationalOperator40/1 -> LogicalOperator32/2
  RelationalOperator51/1 -> LogicalOperator32/1
  Selector/1 -> RelationalOperator/1, RelationalOperator1/1, RelationalOperator2/1, RelationalOperator3/1, RelationalOperator8/1, RelationalOperator9/1, RelationalOperator10/1, RelationalOperator11/1, RelationalOperator16/1, RelationalOperator17/1, RelationalOperator24/1, RelationalOperator25/1, RelationalOperator30/1, RelationalOperator31/1, RelationalOperator18/1, RelationalOperator19/1, RelationalOperator32/1, RelationalOperator33/1, RelationalOperator44/1, RelationalOperator55/1, RelationalOperator62/1, RelationalOperator63/1, RelationalOperator34/1, RelationalOperator35/1, RelationalOperator40/1
  LogicalOperator4/1 -> SERVICE_SUPPORTED/1
  LogicalOperator/1 -> LogicalOperator4/1
  LogicalOperator1/1 -> LogicalOperator4/2
  LogicalOperator2/1 -> LogicalOperator4/3
  LogicalOperator3/1 -> LogicalOperator4/4
  LogicalOperator5/1 -> LogicalOperator4/5
  LogicalOperator6/1 -> LogicalOperator4/6
  LogicalOperator7/1 -> LogicalOperator4/7
  LogicalOperator8/1 -> LogicalOperator4/8
  LogicalOperator9/1 -> LogicalOperator4/9
  LogicalOperator10/1 -> LogicalOperator4/10
  LogicalOperator11/1 -> LogicalOperator4/11
  LogicalOperator12/1 -> LogicalOperator4/12
  LogicalOperator13/1 -> LogicalOperator4/13
  LogicalOperator14/1 -> LogicalOperator4/14
  LogicalOperator15/1 -> LogicalOperator4/15
  LogicalOperator16/1 -> LogicalOperator4/16
  LogicalOperator17/1 -> LogicalOperator4/17
  LogicalOperator18/1 -> LogicalOperator4/18
  LogicalOperator26/1 -> LogicalOperator4/19
  LogicalOperator27/1 -> LogicalOperator4/20
  LogicalOperator28/1 -> LogicalOperator4/21
  LogicalOperator29/1 -> LogicalOperator4/22
  LogicalOperator30/1 -> LogicalOperator4/23
  LogicalOperator31/1 -> LogicalOperator4/24
  LogicalOperator32/1 -> LogicalOperator4/25

== system Renault_Library/Services ECU/Diag/RSA_DiagnosticManagement/DIAGNOSTIC_SANCTION_TOP/GeneralService/Service_Supported_In_Active_Session ==
  DIAG_BUFFER_REQUEST : Inport  OutDataTypeStr=uint8  PortDimensions=4095
  SESSION_ACTIVE : Inport  Port=2  OutDataTypeStr=Enum: TYP_SESSION_ACTIVE
  Constant1 : Constant  Value=TYP_SESSION_ACTIVE.PROGRAMMING_SESSION  OutDataTypeStr=Inherit: Inherit via back propagation  SampleTime=-1
  Constant2 : Constant  Value=TYP_SESSION_ACTIVE.EXTENDED_SESSION  OutDataTypeStr=Inherit: Inherit via back propagation  SampleTime=-1
  Constant3 : Constant  Value=TYP_SESSION_ACTIVE.SAFETY_SYSTEM_SESSION  OutDataTypeStr=Inherit: Inherit via back propagation  SampleTime=-1
  Constant8 : Constant  Value=TYP_SESSION_ACTIVE.DEFAULT_SESSION  OutDataTypeStr=Inherit: Inherit via back propagation  SampleTime=-1
  LogicalOperator101 : Logic  Operator=OR  Inputs=4  OutDataTypeStr=boolean
  RelationalOperator{1..3,9} (4 alike) : RelationalOperator  Operator===  OutDataTypeStr=boolean  InputSameDT=on
  Selector : Selector  InputPortWidth=4095  IndexOptions=Index vector (dialog)  Indices=[1]  OutputSizes=1
  SubsystemDefaultSession : Subsystem
  SubsystemExtendedSession : Subsystem
  SubsystemProgrammingSession : Subsystem
  SubsystemSafetySession : Subsystem
  SERVICE_SUPPORTED_IN_ACTIVE_SESSION : Outport  OutDataTypeStr=boolean
  wires:
  Constant8/1 -> RelationalOperator9/2
  SESSION_ACTIVE/1 -> RelationalOperator9/1, RelationalOperator1/1, RelationalOperator2/1, RelationalOperator3/1
  Constant1/1 -> RelationalOperator1/2
  Constant2/1 -> RelationalOperator2/2
  DIAG_BUFFER_REQUEST/1 -> Selector/1
  LogicalOperator101/1 -> SERVICE_SUPPORTED_IN_ACTIVE_SESSION/1
  Selector/1 -> [Number_Service]
  RelationalOperator9/1 -> SubsystemDefaultSession/1(Active_session)
  RelationalOperator1/1 -> SubsystemProgrammingSession/1(Active_session)
  RelationalOperator2/1 -> SubsystemExtendedSession/1(Active_session)
  Constant3/1 -> RelationalOperator3/2
  RelationalOperator3/1 -> SubsystemSafetySession/1(Active_session)
  SubsystemDefaultSession/1(Out1) -> LogicalOperator101/1
  SubsystemProgrammingSession/1(Out1) -> LogicalOperator101/2
  SubsystemExtendedSession/1(Out1) -> LogicalOperator101/3
  SubsystemSafetySession/1(Out1) -> LogicalOperator101/4
  [Number_Service] -> SubsystemDefaultSession/2(Number_Service)
  [Number_Service] -> SubsystemProgrammingSession/2(Number_Service)
  [Number_Service] -> SubsystemExtendedSession/2(Number_Service)
  [Number_Service] -> SubsystemSafetySession/2(Number_Service)

== system Renault_Library/Services ECU/Diag/RSA_DiagnosticManagement/DIAGNOSTIC_SANCTION_TOP/Service10/Check_Lenght_Message_Service10 ==
  REQ_DATA_NUMBER : Inport
  Constant8 : Constant  Value=2  OutDataTypeStr=Inherit: Inherit via back propagation  SampleTime=-1
  RelationalOperator9 : RelationalOperator  Operator===  OutDataTypeStr=boolean  InputSameDT=on
  LENGTH_MESSAGE_CORRECT : Outport
  wires:
  REQ_DATA_NUMBER/1 -> RelationalOperator9/1
  Constant8/1 -> RelationalOperator9/2
  RelationalOperator9/1 -> LENGTH_MESSAGE_CORRECT/1

== system Renault_Library/Services ECU/Diag/RSA_DiagnosticManagement/DIAGNOSTIC_SANCTION_TOP/Service10/SubFunction_Supported_Service10 ==
  DIAG_BUFFER_REQUEST : Inport
  BitwiseOperator1 : simulink/Logic and Bit Operations/Bitwise Operator  logicop=AND  UseBitMask=on  BitMask=bin2dec('01111111')  BitMaskRealWorld=Stored Integer
  BitwiseOperator4 : simulink/Logic and Bit Operations/Bitwise Operator  logicop=AND  UseBitMask=on  BitMask=bin2dec('10000000')  BitMaskRealWorld=Stored Integer
  Constant : Constant  OutDataTypeStr=Inherit: Inherit via back propagation  SampleTime=-1
  Constant1 : Constant  Value=2  OutDataTypeStr=Inherit: Inherit via back propagation  SampleTime=-1
  Constant10 : Constant  Value=SafetySystemDiagnosticSession  OutDataTypeStr=Inherit: Inherit via back propagation  SampleTime=-1
  Constant{5,7,9,11} (4 alike) : Constant  Value=true  OutDataTypeStr=boolean  SampleTime=-1
  Constant16 : Constant  OutDataTypeStr=Inherit: Inherit via back propagation  SampleTime=-1
  Constant2 : Constant  Value=3  OutDataTypeStr=Inherit: Inherit via back propagation  SampleTime=-1
  Constant3 : Constant  Value=DefaultSession  OutDataTypeStr=Inherit: Inherit via back propagation  SampleTime=-1
  Constant4 : Constant  Value=4  OutDataTypeStr=Inherit: Inherit via back propagation  SampleTime=-1
  Constant6 : Constant  Value=ProgrammingSession  OutDataTypeStr=Inherit: Inherit via back propagation  SampleTime=-1
  Constant8 : Constant  Value=ExtendedDiagnosticSession  OutDataTypeStr=Inherit: Inherit via back propagation  SampleTime=-1
  LogicalOperator{,1..3} (4 alike) : Logic  OutDataTypeStr=boolean
  LogicalOperator4 : Logic  Operator=OR  Inputs=4  OutDataTypeStr=boolean
  RelationalOperator{,1..7,16} (9 alike) : RelationalOperator  Operator===  OutDataTypeStr=boolean  InputSameDT=on
  Selector : Selector  InputPortWidth=4095  IndexOptions=Index vector (dialog)  Indices=[2]  OutputSizes=1
  ShiftArithmetic3 : ArithShift  BitShiftNumber=7
  SUPPRESS_POS_RES : Outport
  SUBFUNCTION_SUPPORTED : Outport  Port=2
  wires:
  DIAG_BUFFER_REQUEST/1 -> Selector/1
  Constant/1 -> RelationalOperator/2
  Constant1/1 -> RelationalOperator1/2
  Constant2/1 -> RelationalOperator2/2
  Constant4/1 -> RelationalOperator3/2
  Constant3/1 -> RelationalOperator4/1
  Constant11/1 -> RelationalOperator4/2
  RelationalOperator/1 -> LogicalOperator/2
  RelationalOperator4/1 -> LogicalOperator/1
  Constant9/1 -> RelationalOperator5/2
  Constant6/1 -> RelationalOperator5/1
  RelationalOperator1/1 -> LogicalOperator1/2
  RelationalOperator5/1 -> LogicalOperator1/1
  Constant7/1 -> RelationalOperator6/2
  Constant8/1 -> RelationalOperator6/1
  RelationalOperator2/1 -> LogicalOperator2/2
  RelationalOperator6/1 -> LogicalOperator2/1
  Constant10/1 -> RelationalOperator7/1
  Constant5/1 -> RelationalOperator7/2
  RelationalOperator7/1 -> LogicalOperator3/1
  LogicalOperator1/1 -> LogicalOperator4/2
  LogicalOperator2/1 -> LogicalOperator4/3
  LogicalOperator/1 -> LogicalOperator4/1
  LogicalOperator3/1 -> LogicalOperator4/4
  LogicalOperator4/1 -> SUBFUNCTION_SUPPORTED/1
  RelationalOperator3/1 -> LogicalOperator3/2
  RelationalOperator16/1 -> SUPPRESS_POS_RES/1
  BitwiseOperator4/1 -> ShiftArithmetic3/1
  ShiftArithmetic3/1 -> RelationalOperator16/1
  Selector/1 -> BitwiseOperator4/1, BitwiseOperator1/1
  Constant16/1 -> RelationalOperator16/2
  BitwiseOperator1/1 -> RelationalOperator/1, RelationalOperator1/1, RelationalOperator2/1, RelationalOperator3/1

== system Renault_Library/Services ECU/Diag/RSA_DiagnosticManagement/DIAGNOSTIC_SANCTION_TOP/Service11/Check_Lenght_Message_Service11 ==
  REQ_DATA_NUMBER : Inport
  Constant1 : Constant  Value=2  OutDataTypeStr=Inherit: Inherit via back propagation  SampleTime=-1
  Constant8 : Constant  Value=4094  OutDataTypeStr=Inherit: Inherit via back propagation  SampleTime=-1
  LogicalOperator : Logic  OutDataTypeStr=boolean
  RelationalOperator1 : RelationalOperator  OutDataTypeStr=boolean  InputSameDT=on
  RelationalOperator9 : RelationalOperator  Operator=<  OutDataTypeStr=boolean  InputSameDT=on
  LENGTH_MESSAGE_CORRECT : Outport
  wires:
  LogicalOperator/1 -> LENGTH_MESSAGE_CORRECT/1
  RelationalOperator1/1 -> LogicalOperator/2
  RelationalOperator9/1 -> LogicalOperator/1
  Constant1/1 -> RelationalOperator1/2
  Constant8/1 -> RelationalOperator9/2
  REQ_DATA_NUMBER/1 -> RelationalOperator1/1, RelationalOperator9/1
